simulink model "SeatClimaCtrlMgr"  (R2022b)
settings: Solver=FixedStepDiscrete  StartTime=0.0  StopTime=10.0  FixedStep=0.1  RelTol=1e-3
settings Model: Creator=blue.ge  LastModifiedBy=blue.ge
settings DataIOCC: SaveFormat=StructureWithTime
settings OptimizationCC: DefaultParameterBehavior=Inlined  EnhancedBackFolding=on  PassReuseOutputArgsAs=Individual arguments  ZeroExternalMemoryAtStartup=off  ZeroInternalMemoryAtStartup=off  OptimizeBlockOrder=speed  DifferentSizesBufferReuse=on  OptimizationCustomize=off  EfficientTunableParamExpr=on
settings DebuggingCC: ParameterTunabilityLossMsg=error
settings ModelReferenceCC: ModelReferenceNumInstancesAllowed=Single
settings SFSimCC: MATLABDynamicMemAlloc=off
settings RTWCC: Description=AUTOSAR  SystemTargetFile=autosar.tlc  GenCodeOnly=on  TemplateMakefile=ert_default_tmf
settings CodeAppCC: ForceParamTrailComments=on  IgnoreCustomStorageClasses=off  OperatorAnnotations=on  SimulinkDataObjDesc=on  SFDataObjDesc=on  InsertBlockDesc=on
settings STFCustomTargetCC: TargetLangStandard=C99 (ISO)  MultiwordLength=256  ParMdlRefBuildCompliant=on  CompOptLevelCompliant=on  IncludeMdlTerminateFcn=off  CombineOutputUpdateFcns=on  SuppressErrorStatus=on  ERTCustomFileBanners=on  SupportNonFinite=off  SupportComplex=off  SupportContinuousTime=off  SupportNonInlinedSFcns=off  RemoveResetFunc=on  MATLABClassNameForMDSCustomization=Simulink.SoftwareTarget.ERTCustomization  AutosarCompliant=on  UseToolchainInfoCompliant=on  LookupTableObjectStructAxisOrder=2,1,3,4,...  LUTObjectStructOrderEvenSpacing=Size,Table,Breakpoints  SystemTargetFile=autosar.tlc
settings ERTTargetCC: TargetLangStandard=C99 (ISO)  ConcurrentExecutionCompliant=off  IncludeMdlTerminateFcn=off  SuppressErrorStatus=on  SupportNonFinite=off  SupportComplex=off  ModelStepFunctionPrototypeControlCompliant=off  CPPClassGenCompliant=off  AutosarCompliant=on  LookupTableObjectStructAxisOrder=2,1,3,4,...  LUTObjectStructOrderEvenSpacing=Size,Table,Breakpoints  InlinedParameterPlacement=NonHierarchical  ERTCustomFileTemplate=example_file_process.tlc

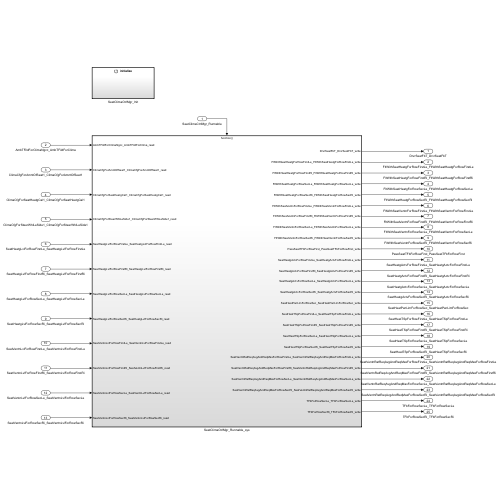
[thumbnail: Simulink's stored preview of the top-level diagram]
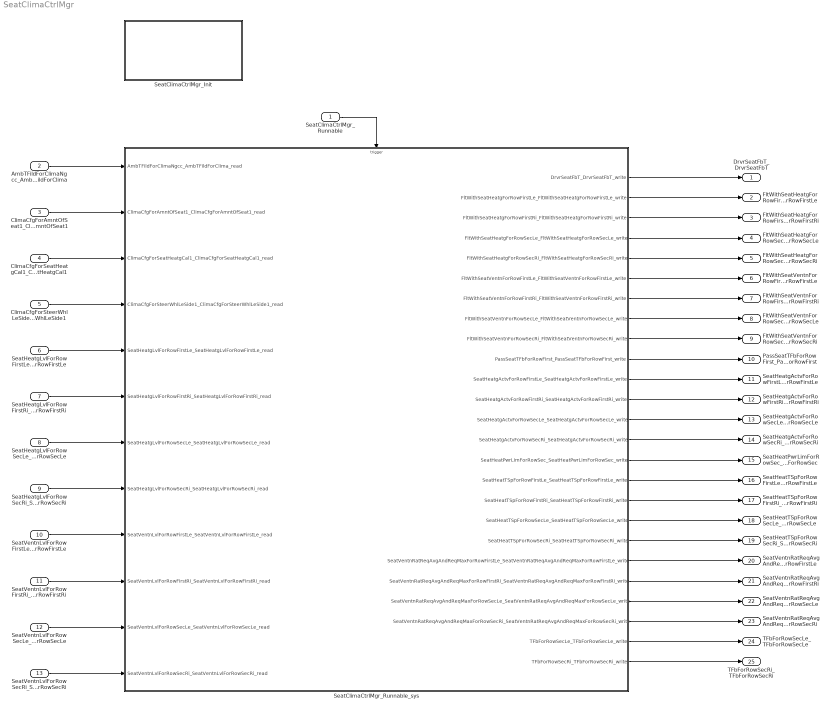
== system SeatClimaCtrlMgr ==
  SeatClimaCtrlMgr_Runnable : Inport  SampleTime=0.1  OutputFunctionCall=on
  AmbTFildForClimaNgcc_AmbTFildForClima : Inport  Port=2  OutDataTypeStr=T30  PortDimensions=1
  ClimaCfgForAmntOfSeat1_ClimaCfgForAmntOfSeat1 : Inport  Port=3  OutDataTypeStr=Enum: ClimaCfgForAmntOfSeat1  PortDimensions=1
  ClimaCfgForSeatHeatgCal1_ClimaCfgForSeatHeatgCal1 : Inport  Port=4  OutDataTypeStr=Enum: CarCfgForSeatHeatgCal1  PortDimensions=1
  ClimaCfgForSteerWhlLeSide1_ClimaCfgForSteerWhlLeSide1 : Inport  Port=5  OutDataTypeStr=Boolean  PortDimensions=1
  SeatHeatgLvlForRowFirstLe_SeatHeatgLvlForRowFirstLe : Inport  Port=6  OutDataTypeStr=Enum: SeatClimaLvl  PortDimensions=1
  SeatHeatgLvlForRowFirstRi_SeatHeatgLvlForRowFirstRi : Inport  Port=7  OutDataTypeStr=Enum: SeatClimaLvl  PortDimensions=1
  SeatHeatgLvlForRowSecLe_SeatHeatgLvlForRowSecLe : Inport  Port=8  OutDataTypeStr=Enum: SeatClimaLvl  PortDimensions=1
  SeatHeatgLvlForRowSecRi_SeatHeatgLvlForRowSecRi : Inport  Port=9  OutDataTypeStr=Enum: SeatClimaLvl  PortDimensions=1
  SeatVentnLvlForRowFirstLe_SeatVentnLvlForRowFirstLe : Inport  Port=10  OutDataTypeStr=Enum: SeatClimaLvl  PortDimensions=1
  SeatVentnLvlForRowFirstRi_SeatVentnLvlForRowFirstRi : Inport  Port=11  OutDataTypeStr=Enum: SeatClimaLvl  PortDimensions=1
  SeatVentnLvlForRowSecLe_SeatVentnLvlForRowSecLe : Inport  Port=12  OutDataTypeStr=Enum: SeatClimaLvl  PortDimensions=1
  SeatVentnLvlForRowSecRi_SeatVentnLvlForRowSecRi : Inport  Port=13  OutDataTypeStr=Enum: SeatClimaLvl  PortDimensions=1
  SeatClimaCtrlMgr_Init : Subsystem
  SeatClimaCtrlMgr_Runnable_sys : Subsystem
  DrvrSeatFbT_DrvrSeatFbT : Outport  OutDataTypeStr=T30  PortDimensions=1
  FltWithSeatHeatgForRowFirstLe_FltWithSeatHeatgForRowFirstLe : Outport  Port=2  OutDataTypeStr=Boolean  PortDimensions=1
  FltWithSeatHeatgForRowFirstRi_FltWithSeatHeatgForRowFirstRi : Outport  Port=3  OutDataTypeStr=Boolean  PortDimensions=1
  FltWithSeatHeatgForRowSecLe_FltWithSeatHeatgForRowSecLe : Outport  Port=4  OutDataTypeStr=Boolean  PortDimensions=1
  FltWithSeatHeatgForRowSecRi_FltWithSeatHeatgForRowSecRi : Outport  Port=5  OutDataTypeStr=Boolean  PortDimensions=1
  FltWithSeatVentnForRowFirstLe_FltWithSeatVentnForRowFirstLe : Outport  Port=6  OutDataTypeStr=Boolean  PortDimensions=1
  FltWithSeatVentnForRowFirstRi_FltWithSeatVentnForRowFirstRi : Outport  Port=7  OutDataTypeStr=Boolean  PortDimensions=1
  FltWithSeatVentnForRowSecLe_FltWithSeatVentnForRowSecLe : Outport  Port=8  OutDataTypeStr=Boolean  PortDimensions=1
  FltWithSeatVentnForRowSecRi_FltWithSeatVentnForRowSecRi : Outport  Port=9  OutDataTypeStr=Boolean  PortDimensions=1
  PassSeatTFbForRowFirst_PassSeatTFbForRowFirst : Outport  Port=10  OutDataTypeStr=T30  PortDimensions=1
  SeatHeatgActvForRowFirstLe_SeatHeatgActvForRowFirstLe : Outport  Port=11  OutDataTypeStr=Boolean  PortDimensions=1
  SeatHeatgActvForRowFirstRi_SeatHeatgActvForRowFirstRi : Outport  Port=12  OutDataTypeStr=Boolean  PortDimensions=1
  SeatHeatgActvForRowSecLe_SeatHeatgActvForRowSecLe : Outport  Port=13  OutDataTypeStr=Boolean  PortDimensions=1
  SeatHeatgActvForRowSecRi_SeatHeatgActvForRowSecRi : Outport  Port=14  OutDataTypeStr=Boolean  PortDimensions=1
  SeatHeatPwrLimForRowSec_SeatHeatPwrLimForRowSec : Outport  Port=15  OutDataTypeStr=Perc30  PortDimensions=1
  SeatHeatTSpForRowFirstLe_SeatHeatTSpForRowFirstLe : Outport  Port=16  OutDataTypeStr=T30  PortDimensions=1
  SeatHeatTSpForRowFirstRi_SeatHeatTSpForRowFirstRi : Outport  Port=17  OutDataTypeStr=T30  PortDimensions=1
  SeatHeatTSpForRowSecLe_SeatHeatTSpForRowSecLe : Outport  Port=18  OutDataTypeStr=T30  PortDimensions=1
  SeatHeatTSpForRowSecRi_SeatHeatTSpForRowSecRi : Outport  Port=19  OutDataTypeStr=T30  PortDimensions=1
  SeatVentnRatReqAvgAndReqMaxForRowFirstLe_SeatVentnRatReqAvgAndReqMaxForRowFirstLe : Outport  Port=20  OutDataTypeStr=Fac30  PortDimensions=1
  SeatVentnRatReqAvgAndReqMaxForRowFirstRi_SeatVentnRatReqAvgAndReqMaxForRowFirstRi : Outport  Port=21  OutDataTypeStr=Fac30  PortDimensions=1
  SeatVentnRatReqAvgAndReqMaxForRowSecLe_SeatVentnRatReqAvgAndReqMaxForRowSecLe : Outport  Port=22  OutDataTypeStr=Fac30  PortDimensions=1
  SeatVentnRatReqAvgAndReqMaxForRowSecRi_SeatVentnRatReqAvgAndReqMaxForRowSecRi : Outport  Port=23  OutDataTypeStr=Fac30  PortDimensions=1
  TFbForRowSecLe_TFbForRowSecLe : Outport  Port=24  OutDataTypeStr=T30  PortDimensions=1
  TFbForRowSecRi_TFbForRowSecRi : Outport  Port=25  OutDataTypeStr=T30  PortDimensions=1
  wires:
  AmbTFildForClimaNgcc_AmbTFildForClima/1 -> SeatClimaCtrlMgr_Runnable_sys/1(AmbTFildForClimaNgcc_AmbTFildForClima_read)
  ClimaCfgForAmntOfSeat1_ClimaCfgForAmntOfSeat1/1 -> SeatClimaCtrlMgr_Runnable_sys/2(ClimaCfgForAmntOfSeat1_ClimaCfgForAmntOfSeat1_read)
  ClimaCfgForSeatHeatgCal1_ClimaCfgForSeatHeatgCal1/1 -> SeatClimaCtrlMgr_Runnable_sys/3(ClimaCfgForSeatHeatgCal1_ClimaCfgForSeatHeatgCal1_read)
  ClimaCfgForSteerWhlLeSide1_ClimaCfgForSteerWhlLeSide1/1 -> SeatClimaCtrlMgr_Runnable_sys/4(ClimaCfgForSteerWhlLeSide1_ClimaCfgForSteerWhlLeSide1_read)
  SeatHeatgLvlForRowFirstLe_SeatHeatgLvlForRowFirstLe/1 -> SeatClimaCtrlMgr_Runnable_sys/5(SeatHeatgLvlForRowFirstLe_SeatHeatgLvlForRowFirstLe_read)
  SeatHeatgLvlForRowFirstRi_SeatHeatgLvlForRowFirstRi/1 -> SeatClimaCtrlMgr_Runnable_sys/6(SeatHeatgLvlForRowFirstRi_SeatHeatgLvlForRowFirstRi_read)
  SeatHeatgLvlForRowSecLe_SeatHeatgLvlForRowSecLe/1 -> SeatClimaCtrlMgr_Runnable_sys/7(SeatHeatgLvlForRowSecLe_SeatHeatgLvlForRowSecLe_read)
  SeatHeatgLvlForRowSecRi_SeatHeatgLvlForRowSecRi/1 -> SeatClimaCtrlMgr_Runnable_sys/8(SeatHeatgLvlForRowSecRi_SeatHeatgLvlForRowSecRi_read)
  SeatVentnLvlForRowFirstLe_SeatVentnLvlForRowFirstLe/1 -> SeatClimaCtrlMgr_Runnable_sys/9(SeatVentnLvlForRowFirstLe_SeatVentnLvlForRowFirstLe_read)
  SeatVentnLvlForRowFirstRi_SeatVentnLvlForRowFirstRi/1 -> SeatClimaCtrlMgr_Runnable_sys/10(SeatVentnLvlForRowFirstRi_SeatVentnLvlForRowFirstRi_read)
  SeatVentnLvlForRowSecLe_SeatVentnLvlForRowSecLe/1 -> SeatClimaCtrlMgr_Runnable_sys/11(SeatVentnLvlForRowSecLe_SeatVentnLvlForRowSecLe_read)
  SeatVentnLvlForRowSecRi_SeatVentnLvlForRowSecRi/1 -> SeatClimaCtrlMgr_Runnable_sys/12(SeatVentnLvlForRowSecRi_SeatVentnLvlForRowSecRi_read)
  SeatClimaCtrlMgr_Runnable_sys/1(DrvrSeatFbT_DrvrSeatFbT_write) -> DrvrSeatFbT_DrvrSeatFbT/1
  SeatClimaCtrlMgr_Runnable_sys/2(FltWithSeatHeatgForRowFirstLe_FltWithSeatHeatgForRowFirstLe_write) -> FltWithSeatHeatgForRowFirstLe_FltWithSeatHeatgForRowFirstLe/1
  SeatClimaCtrlMgr_Runnable_sys/3(FltWithSeatHeatgForRowFirstRi_FltWithSeatHeatgForRowFirstRi_write) -> FltWithSeatHeatgForRowFirstRi_FltWithSeatHeatgForRowFirstRi/1
  SeatClimaCtrlMgr_Runnable_sys/4(FltWithSeatHeatgForRowSecLe_FltWithSeatHeatgForRowSecLe_write) -> FltWithSeatHeatgForRowSecLe_FltWithSeatHeatgForRowSecLe/1
  SeatClimaCtrlMgr_Runnable_sys/5(FltWithSeatHeatgForRowSecRi_FltWithSeatHeatgForRowSecRi_write) -> FltWithSeatHeatgForRowSecRi_FltWithSeatHeatgForRowSecRi/1
  SeatClimaCtrlMgr_Runnable_sys/6(FltWithSeatVentnForRowFirstLe_FltWithSeatVentnForRowFirstLe_write) -> FltWithSeatVentnForRowFirstLe_FltWithSeatVentnForRowFirstLe/1
  SeatClimaCtrlMgr_Runnable_sys/7(FltWithSeatVentnForRowFirstRi_FltWithSeatVentnForRowFirstRi_write) -> FltWithSeatVentnForRowFirstRi_FltWithSeatVentnForRowFirstRi/1
  SeatClimaCtrlMgr_Runnable_sys/8(FltWithSeatVentnForRowSecLe_FltWithSeatVentnForRowSecLe_write) -> FltWithSeatVentnForRowSecLe_FltWithSeatVentnForRowSecLe/1
  SeatClimaCtrlMgr_Runnable_sys/9(FltWithSeatVentnForRowSecRi_FltWithSeatVentnForRowSecRi_write) -> FltWithSeatVentnForRowSecRi_FltWithSeatVentnForRowSecRi/1
  SeatClimaCtrlMgr_Runnable_sys/10(PassSeatTFbForRowFirst_PassSeatTFbForRowFirst_write) -> PassSeatTFbForRowFirst_PassSeatTFbForRowFirst/1
  SeatClimaCtrlMgr_Runnable_sys/11(SeatHeatgActvForRowFirstLe_SeatHeatgActvForRowFirstLe_write) -> SeatHeatgActvForRowFirstLe_SeatHeatgActvForRowFirstLe/1
  SeatClimaCtrlMgr_Runnable_sys/12(SeatHeatgActvForRowFirstRi_SeatHeatgActvForRowFirstRi_write) -> SeatHeatgActvForRowFirstRi_SeatHeatgActvForRowFirstRi/1
  SeatClimaCtrlMgr_Runnable_sys/13(SeatHeatgActvForRowSecLe_SeatHeatgActvForRowSecLe_write) -> SeatHeatgActvForRowSecLe_SeatHeatgActvForRowSecLe/1
  SeatClimaCtrlMgr_Runnable_sys/14(SeatHeatgActvForRowSecRi_SeatHeatgActvForRowSecRi_write) -> SeatHeatgActvForRowSecRi_SeatHeatgActvForRowSecRi/1
  SeatClimaCtrlMgr_Runnable_sys/15(SeatHeatPwrLimForRowSec_SeatHeatPwrLimForRowSec_write) -> SeatHeatPwrLimForRowSec_SeatHeatPwrLimForRowSec/1
  SeatClimaCtrlMgr_Runnable_sys/16(SeatHeatTSpForRowFirstLe_SeatHeatTSpForRowFirstLe_write) -> SeatHeatTSpForRowFirstLe_SeatHeatTSpForRowFirstLe/1
  SeatClimaCtrlMgr_Runnable_sys/17(SeatHeatTSpForRowFirstRi_SeatHeatTSpForRowFirstRi_write) -> SeatHeatTSpForRowFirstRi_SeatHeatTSpForRowFirstRi/1
  SeatClimaCtrlMgr_Runnable_sys/18(SeatHeatTSpForRowSecLe_SeatHeatTSpForRowSecLe_write) -> SeatHeatTSpForRowSecLe_SeatHeatTSpForRowSecLe/1
  SeatClimaCtrlMgr_Runnable_sys/19(SeatHeatTSpForRowSecRi_SeatHeatTSpForRowSecRi_write) -> SeatHeatTSpForRowSecRi_SeatHeatTSpForRowSecRi/1
  SeatClimaCtrlMgr_Runnable_sys/20(SeatVentnRatReqAvgAndReqMaxForRowFirstLe_SeatVentnRatReqAvgAndReqMaxForRowFirstLe_write) -> SeatVentnRatReqAvgAndReqMaxForRowFirstLe_SeatVentnRatReqAvgAndReqMaxForRowFirstLe/1
  SeatClimaCtrlMgr_Runnable_sys/21(SeatVentnRatReqAvgAndReqMaxForRowFirstRi_SeatVentnRatReqAvgAndReqMaxForRowFirstRi_write) -> SeatVentnRatReqAvgAndReqMaxForRowFirstRi_SeatVentnRatReqAvgAndReqMaxForRowFirstRi/1
  SeatClimaCtrlMgr_Runnable_sys/22(SeatVentnRatReqAvgAndReqMaxForRowSecLe_SeatVentnRatReqAvgAndReqMaxForRowSecLe_write) -> SeatVentnRatReqAvgAndReqMaxForRowSecLe_SeatVentnRatReqAvgAndReqMaxForRowSecLe/1
  SeatClimaCtrlMgr_Runnable_sys/23(SeatVentnRatReqAvgAndReqMaxForRowSecRi_SeatVentnRatReqAvgAndReqMaxForRowSecRi_write) -> SeatVentnRatReqAvgAndReqMaxForRowSecRi_SeatVentnRatReqAvgAndReqMaxForRowSecRi/1
  SeatClimaCtrlMgr_Runnable_sys/24(TFbForRowSecLe_TFbForRowSecLe_write) -> TFbForRowSecLe_TFbForRowSecLe/1
  SeatClimaCtrlMgr_Runnable_sys/25(TFbForRowSecRi_TFbForRowSecRi_write) -> TFbForRowSecRi_TFbForRowSecRi/1
  SeatClimaCtrlMgr_Runnable/1 -> SeatClimaCtrlMgr_Runnable_sys/Trigger

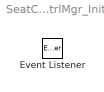
== system SeatClimaCtrlMgr/SeatClimaCtrlMgr_Init ==
  Event Listener : EventListener  EventName=reset

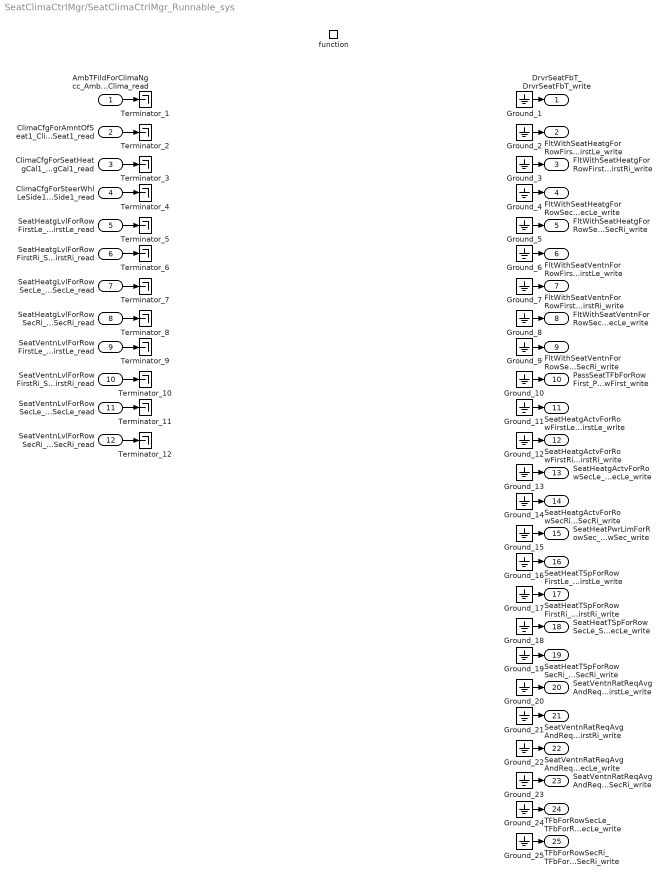
== system SeatClimaCtrlMgr/SeatClimaCtrlMgr_Runnable_sys ==
  AmbTFildForClimaNgcc_AmbTFildForClima_read : Inport
  ClimaCfgForAmntOfSeat1_ClimaCfgForAmntOfSeat1_read : Inport  Port=2
  ClimaCfgForSeatHeatgCal1_ClimaCfgForSeatHeatgCal1_read : Inport  Port=3
  ClimaCfgForSteerWhlLeSide1_ClimaCfgForSteerWhlLeSide1_read : Inport  Port=4
  SeatHeatgLvlForRowFirstLe_SeatHeatgLvlForRowFirstLe_read : Inport  Port=5
  SeatHeatgLvlForRowFirstRi_SeatHeatgLvlForRowFirstRi_read : Inport  Port=6
  SeatHeatgLvlForRowSecLe_SeatHeatgLvlForRowSecLe_read : Inport  Port=7
  SeatHeatgLvlForRowSecRi_SeatHeatgLvlForRowSecRi_read : Inport  Port=8
  SeatVentnLvlForRowFirstLe_SeatVentnLvlForRowFirstLe_read : Inport  Port=9
  SeatVentnLvlForRowFirstRi_SeatVentnLvlForRowFirstRi_read : Inport  Port=10
  SeatVentnLvlForRowSecLe_SeatVentnLvlForRowSecLe_read : Inport  Port=11
  SeatVentnLvlForRowSecRi_SeatVentnLvlForRowSecRi_read : Inport  Port=12
  function : TriggerPort  TriggerType=function-call  VariantControl=(inherit)  SampleTimeType=periodic  SampleTime=-1
  Ground_{1..25} (25 alike) : Ground
  Terminator_{1..12} (12 alike) : Terminator
  DrvrSeatFbT_DrvrSeatFbT_write : Outport
      settings: EnsureOutportIsVirtual=on
  FltWithSeatHeatgForRowFirstLe_FltWithSeatHeatgForRowFirstLe_write : Outport  Port=2
      settings: EnsureOutportIsVirtual=on
  FltWithSeatHeatgForRowFirstRi_FltWithSeatHeatgForRowFirstRi_write : Outport  Port=3
      settings: EnsureOutportIsVirtual=on
  FltWithSeatHeatgForRowSecLe_FltWithSeatHeatgForRowSecLe_write : Outport  Port=4
      settings: EnsureOutportIsVirtual=on
  FltWithSeatHeatgForRowSecRi_FltWithSeatHeatgForRowSecRi_write : Outport  Port=5
      settings: EnsureOutportIsVirtual=on
  FltWithSeatVentnForRowFirstLe_FltWithSeatVentnForRowFirstLe_write : Outport  Port=6
      settings: EnsureOutportIsVirtual=on
  FltWithSeatVentnForRowFirstRi_FltWithSeatVentnForRowFirstRi_write : Outport  Port=7
      settings: EnsureOutportIsVirtual=on
  FltWithSeatVentnForRowSecLe_FltWithSeatVentnForRowSecLe_write : Outport  Port=8
      settings: EnsureOutportIsVirtual=on
  FltWithSeatVentnForRowSecRi_FltWithSeatVentnForRowSecRi_write : Outport  Port=9
      settings: EnsureOutportIsVirtual=on
  PassSeatTFbForRowFirst_PassSeatTFbForRowFirst_write : Outport  Port=10
      settings: EnsureOutportIsVirtual=on
  SeatHeatgActvForRowFirstLe_SeatHeatgActvForRowFirstLe_write : Outport  Port=11
      settings: EnsureOutportIsVirtual=on
  SeatHeatgActvForRowFirstRi_SeatHeatgActvForRowFirstRi_write : Outport  Port=12
      settings: EnsureOutportIsVirtual=on
  SeatHeatgActvForRowSecLe_SeatHeatgActvForRowSecLe_write : Outport  Port=13
      settings: EnsureOutportIsVirtual=on
  SeatHeatgActvForRowSecRi_SeatHeatgActvForRowSecRi_write : Outport  Port=14
      settings: EnsureOutportIsVirtual=on
  SeatHeatPwrLimForRowSec_SeatHeatPwrLimForRowSec_write : Outport  Port=15
      settings: EnsureOutportIsVirtual=on
  SeatHeatTSpForRowFirstLe_SeatHeatTSpForRowFirstLe_write : Outport  Port=16
      settings: EnsureOutportIsVirtual=on
  SeatHeatTSpForRowFirstRi_SeatHeatTSpForRowFirstRi_write : Outport  Port=17
      settings: EnsureOutportIsVirtual=on
  SeatHeatTSpForRowSecLe_SeatHeatTSpForRowSecLe_write : Outport  Port=18
      settings: EnsureOutportIsVirtual=on
  SeatHeatTSpForRowSecRi_SeatHeatTSpForRowSecRi_write : Outport  Port=19
      settings: EnsureOutportIsVirtual=on
  SeatVentnRatReqAvgAndReqMaxForRowFirstLe_SeatVentnRatReqAvgAndReqMaxForRowFirstLe_write : Outport  Port=20
      settings: EnsureOutportIsVirtual=on
  SeatVentnRatReqAvgAndReqMaxForRowFirstRi_SeatVentnRatReqAvgAndReqMaxForRowFirstRi_write : Outport  Port=21
      settings: EnsureOutportIsVirtual=on
  SeatVentnRatReqAvgAndReqMaxForRowSecLe_SeatVentnRatReqAvgAndReqMaxForRowSecLe_write : Outport  Port=22
      settings: EnsureOutportIsVirtual=on
  SeatVentnRatReqAvgAndReqMaxForRowSecRi_SeatVentnRatReqAvgAndReqMaxForRowSecRi_write : Outport  Port=23
      settings: EnsureOutportIsVirtual=on
  TFbForRowSecLe_TFbForRowSecLe_write : Outport  Port=24
      settings: EnsureOutportIsVirtual=on
  TFbForRowSecRi_TFbForRowSecRi_write : Outport  Port=25
      settings: EnsureOutportIsVirtual=on
  wires:
  AmbTFildForClimaNgcc_AmbTFildForClima_read/1 -> Terminator_1/1
  ClimaCfgForAmntOfSeat1_ClimaCfgForAmntOfSeat1_read/1 -> Terminator_2/1
  ClimaCfgForSeatHeatgCal1_ClimaCfgForSeatHeatgCal1_read/1 -> Terminator_3/1
  ClimaCfgForSteerWhlLeSide1_ClimaCfgForSteerWhlLeSide1_read/1 -> Terminator_4/1
  SeatHeatgLvlForRowFirstLe_SeatHeatgLvlForRowFirstLe_read/1 -> Terminator_5/1
  SeatHeatgLvlForRowFirstRi_SeatHeatgLvlForRowFirstRi_read/1 -> Terminator_6/1
  SeatHeatgLvlForRowSecLe_SeatHeatgLvlForRowSecLe_read/1 -> Terminator_7/1
  SeatHeatgLvlForRowSecRi_SeatHeatgLvlForRowSecRi_read/1 -> Terminator_8/1
  SeatVentnLvlForRowFirstLe_SeatVentnLvlForRowFirstLe_read/1 -> Terminator_9/1
  SeatVentnLvlForRowFirstRi_SeatVentnLvlForRowFirstRi_read/1 -> Terminator_10/1
  SeatVentnLvlForRowSecLe_SeatVentnLvlForRowSecLe_read/1 -> Terminator_11/1
  SeatVentnLvlForRowSecRi_SeatVentnLvlForRowSecRi_read/1 -> Terminator_12/1
  Ground_1/1 -> DrvrSeatFbT_DrvrSeatFbT_write/1
  Ground_2/1 -> FltWithSeatHeatgForRowFirstLe_FltWithSeatHeatgForRowFirstLe_write/1
  Ground_3/1 -> FltWithSeatHeatgForRowFirstRi_FltWithSeatHeatgForRowFirstRi_write/1
  Ground_4/1 -> FltWithSeatHeatgForRowSecLe_FltWithSeatHeatgForRowSecLe_write/1
  Ground_5/1 -> FltWithSeatHeatgForRowSecRi_FltWithSeatHeatgForRowSecRi_write/1
  Ground_6/1 -> FltWithSeatVentnForRowFirstLe_FltWithSeatVentnForRowFirstLe_write/1
  Ground_7/1 -> FltWithSeatVentnForRowFirstRi_FltWithSeatVentnForRowFirstRi_write/1
  Ground_8/1 -> FltWithSeatVentnForRowSecLe_FltWithSeatVentnForRowSecLe_write/1
  Ground_9/1 -> FltWithSeatVentnForRowSecRi_FltWithSeatVentnForRowSecRi_write/1
  Ground_10/1 -> PassSeatTFbForRowFirst_PassSeatTFbForRowFirst_write/1
  Ground_11/1 -> SeatHeatgActvForRowFirstLe_SeatHeatgActvForRowFirstLe_write/1
  Ground_12/1 -> SeatHeatgActvForRowFirstRi_SeatHeatgActvForRowFirstRi_write/1
  Ground_13/1 -> SeatHeatgActvForRowSecLe_SeatHeatgActvForRowSecLe_write/1
  Ground_14/1 -> SeatHeatgActvForRowSecRi_SeatHeatgActvForRowSecRi_write/1
  Ground_15/1 -> SeatHeatPwrLimForRowSec_SeatHeatPwrLimForRowSec_write/1
  Ground_16/1 -> SeatHeatTSpForRowFirstLe_SeatHeatTSpForRowFirstLe_write/1
  Ground_17/1 -> SeatHeatTSpForRowFirstRi_SeatHeatTSpForRowFirstRi_write/1
  Ground_18/1 -> SeatHeatTSpForRowSecLe_SeatHeatTSpForRowSecLe_write/1
  Ground_19/1 -> SeatHeatTSpForRowSecRi_SeatHeatTSpForRowSecRi_write/1
  Ground_20/1 -> SeatVentnRatReqAvgAndReqMaxForRowFirstLe_SeatVentnRatReqAvgAndReqMaxForRowFirstLe_write/1
  Ground_21/1 -> SeatVentnRatReqAvgAndReqMaxForRowFirstRi_SeatVentnRatReqAvgAndReqMaxForRowFirstRi_write/1
  Ground_22/1 -> SeatVentnRatReqAvgAndReqMaxForRowSecLe_SeatVentnRatReqAvgAndReqMaxForRowSecLe_write/1
  Ground_23/1 -> SeatVentnRatReqAvgAndReqMaxForRowSecRi_SeatVentnRatReqAvgAndReqMaxForRowSecRi_write/1
  Ground_24/1 -> TFbForRowSecLe_TFbForRowSecLe_write/1
  Ground_25/1 -> TFbForRowSecRi_TFbForRowSecRi_write/1
